simulink model "wlan_phy_rx_pmd"  (Simulink 7.8)
settings: Solver=VariableStepDiscrete  SolverMode=SingleTasking  StartTime=0.0  StopTime=rx_sim_time  RelTol=1e-3
PostLoadFcn:
  | wlan_phy_rx_init
InitFcn:
  | wlan_phy_rx_init
StartFcn:
  | wlan_phy_rx_init
values from neighbouring files (symbols this model uses):
  barker_seq20 = 0.95 * circshift(barker_seq20, [0 4]) ./ max(abs(barker_seq20))    [wlan_phy_rx_init.m]
  bit_scrambler_lfsr_bytes = sum(bit_scrambler_lfsr_bytes .* repmat(2.^[0:7], 127, 1),2)    [wlan_phy_rx_init.m]
  deinterleave_ROM = [deinterleave_ROM interleave_11n_64QAM zeros(1, 512-length(interleave_11n_64QAM))]    [wlan_phy_rx_init.m]
  longCorr_coef_i = [3*real(longCorr_coef)]    [wlan_phy_rx_init.m]
  longCorr_coef_q = [3*imag(longCorr_coef)]    [wlan_phy_rx_init.m]
  MAX_CP_LEN = 32    [wlan_phy_rx_init.m]
  MAX_NCBPS = NCBPS_11n_64QAM    (= 312)    [wlan_phy_rx_init.m]
  MAX_NUM_BYTES = 2^16-1    (= 65535)    [wlan_phy_rx_init.m]
  MAX_NUM_SC = 64    [wlan_phy_rx_init.m]
  MAX_NUM_SYMS = 600    [wlan_phy_rx_init.m]
  NCBPS_11a_BPSK = 48    [wlan_phy_rx_init.m]
  NDBPS_11a_BPSK12 = 24    [wlan_phy_rx_init.m]
  PHY_SIGNAL_MIN_LEN = 14    [wlan_phy_rx_init.m]
  preFFT_sampBuff_numSamps = 4*MAX_NUM_SC    (= 256)    [wlan_phy_rx_init.m]
  rx_sim_sig_samp_time = 8    [wlan_phy_rx_init.m]
  scr_ind_rev = zeros(1,128)    [wlan_phy_rx_init.m]
undefined symbols (not defined in the file or in its folder): gcbh, mcs_rom_11ag, mcs_rom_11n, xlBlockLoadCallback, xlGetNormalizedPeriod, xlipgetpartsetting
libraries: xbsIndex_r4 x3862, simulink x1, xbsEDKLib_r4 x1
(part 9 of 23)

== system wlan_phy_rx_pmd/DSSS Rx/Demod/DBPSK Demod ==
  IQ Valid : Inport
  I : Inport  Port=2
  Q : Inport  Port=3
  Absolute : xbsIndex_r4/Absolute  precision=Full  arith_type=Unsigned  n_bits=16  bin_pt=14  quantization=Truncate  overflow=Wrap  en=off  latency=0  dbl_ovrd=off  block_type=abs
  AddSub : xbsIndex_r4/AddSub  mode=Subtraction  use_carryin=off  use_carryout=off  en=off  latency=0  precision=Full  arith_type=Unsigned  n_bits=16  bin_pt=14  quantization=Truncate  overflow=Wrap  dbl_ovrd=off  use_behavioral_HDL=off  hw_selection=Fabric  pipelined=off  block_type=addsub
  "CORDIC 5.0 " : xbsIndex_r4/CORDIC 5.0   functional_selection=Arc_Tan  architectural_configuration=Parallel  pipelining_mode=Maximum  data_format=SignedFraction  phase_format=Scaled_Radians  input_width=16  output_width=16  round_mode=Truncate  iterations=0  precision=0  compensation_scaling=No_Scale_Compensation  coarse_rotation=on  aclken=off  aresetn=off  out_tready=off  cartesian_has_tuser=off  cartesian_has_tlast=off  cartesian_tuser_width=1  phase_has_tuser=off  phase_has_tlast=off  phase_tuser_width=1  out_tlast_behv=Null  flow_control=NonBlocking  optimize_goal=Resources  trim_axipin_name=on  ip_name=CORDIC  ip_version=5.0  dsptool_ready=true  ipcore_usecache=true  ipcore_useipmodelcache=true  wrapper_available=true  port_translation_map={ 'aclken' => 'en', 'aresetn' => 'rst' }  block_type=cordic_v5_0
      settings: infoedit=CORDIC 5.0
  Constant : xbsIndex_r4/Constant  const=0.5  gui_display_data_type=Fixed-point  arith_type=Signed (2's comp)  n_bits=4  bin_pt=3  preci_type=Single  exp_width=8  frac_width=24  explicit_period=off  period=1  equ=P=C  opselect=C  inp2=PCIN>>17  opr=+  inp1=P  carry=CIN  dbl_ovrd=off  block_type=constant
  Constant1 : xbsIndex_r4/Constant  const=1.5  gui_display_data_type=Fixed-point  arith_type=Signed (2's comp)  n_bits=6  bin_pt=3  preci_type=Single  exp_width=8  frac_width=24  explicit_period=off  period=1  equ=P=C  opselect=C  inp2=PCIN>>17  opr=+  inp1=P  carry=CIN  dbl_ovrd=off  block_type=constant
  Convert : xbsIndex_r4/Convert  gui_display_data_type=Fixed-point  arith_type=Unsigned  n_bits=1  bin_pt=0  float_type=Single  exp_bits=8  fraction_bits=24  quantization=Truncate  overflow=Wrap  en=off  latency=0  dbl_ovrd=off  pipeline=off  block_type=convert
  Gateway Out2 : xbsIndex_r4/Gateway Out  inherit_from_input=off  hdl_port=off  timing_constraint=None  locs_specified=off  LOCs={}  UseAsDAC=off  DACChannel='1'  block_type=gatewayout
  Gateway Out3 : xbsIndex_r4/Gateway Out  inherit_from_input=off  hdl_port=off  timing_constraint=None  locs_specified=off  LOCs={}  UseAsDAC=off  DACChannel='1'  block_type=gatewayout
  Gateway Out6 : xbsIndex_r4/Gateway Out  inherit_from_input=off  hdl_port=off  timing_constraint=None  locs_specified=off  LOCs={}  UseAsDAC=off  DACChannel='1'  block_type=gatewayout
  Logical : xbsIndex_r4/Logical  logical_function=AND  inputs=2  en=off  latency=0  precision=Full  arith_type=Unsigned  n_bits=16  bin_pt=0  align_bp=on  dbl_ovrd=off  block_type=logical
  Phase Cmp : Scope  NumInputPorts=7
      settings: TimeRange=80000  YMin=-1~-0.5~0~-0.1~5~0~0  YMax=1~0.3~1~1.1~40~1~300  SaveName=ScopeData6
  Register{,1..3} (4 alike) : xbsIndex_r4/Register  init=0  rst=off  en=off  dbl_ovrd=off  block_type=register
  Register4 : xbsIndex_r4/Register  init=0  rst=off  en=on  dbl_ovrd=off  block_type=register
  Relational : xbsIndex_r4/Relational  mode=a>b  en=off  latency=0  dbl_ovrd=off  block_type=relational
  Relational1 : xbsIndex_r4/Relational  mode=a<b  en=off  latency=0  dbl_ovrd=off  block_type=relational
  bit : Outport
  bit valid : Outport  Port=2
  wires:
  IQ Valid/1 -> "CORDIC 5.0 "/1
  I/1 -> "CORDIC 5.0 "/2
  Q/1 -> "CORDIC 5.0 "/3
  "CORDIC 5.0 "/2 -> Register1/1
  "CORDIC 5.0 "/1 -> Register/1
  Register4/1 -> AddSub/2, Gateway Out3/1
  AddSub/1 -> Absolute/1
  Constant/1 -> Relational/2
  Absolute/1 -> Gateway Out6/1, Relational/1, Relational1/1
  Relational/1 -> Logical/1
  Convert/1 -> Register2/1
  Gateway Out2/1 -> Phase Cmp/1
  Gateway Out3/1 -> Phase Cmp/2
  Gateway Out6/1 -> Phase Cmp/3
  Constant1/1 -> Relational1/2
  Relational1/1 -> Logical/2
  Logical/1 -> Convert/1
  Register/1 -> Register3/1, Register4/2
  Register1/1 -> AddSub/1, Gateway Out2/1, Register4/1
  Register2/1 -> bit/1
  Register3/1 -> bit valid/1

== system wlan_phy_rx_pmd/DSSS Rx/Demod/De-spread ==
  I : Inport
  Q : Inport  Port=2
  Sym Start : Inport  Port=3
  Accumulator : xbsIndex_r4/Accumulator  operation=Add  n_bits=22  overflow=Wrap  scale=1  rst=on  infoeditControl=reset for floating point data type must be asserted for a minimum of 2 cycles  hasbypass=on  en=off  latency=0  dbl_ovrd=off  msb_inp=100  msb=100  lsb=-100  use_behavioral_HDL=on  implementation=Fabric  block_type=accum
  Accumulator1 : xbsIndex_r4/Accumulator  operation=Add  n_bits=22  overflow=Wrap  scale=1  rst=on  infoeditControl=reset for floating point data type must be asserted for a minimum of 2 cycles  hasbypass=on  en=off  latency=0  dbl_ovrd=off  msb_inp=100  msb=100  lsb=-100  use_behavioral_HDL=on  implementation=Fabric  block_type=accum
  Constant : xbsIndex_r4/Constant  const=0  gui_display_data_type=Fixed-point  arith_type=Unsigned  n_bits=2  bin_pt=0  preci_type=Single  exp_width=8  frac_width=24  explicit_period=off  period=1  equ=P=C  opselect=C  inp2=PCIN>>17  opr=+  inp1=P  carry=CIN  dbl_ovrd=off  block_type=constant
  Constant1 : xbsIndex_r4/Constant  const=0  gui_display_data_type=Boolean  arith_type=Boolean  n_bits=16  bin_pt=14  preci_type=Single  exp_width=8  frac_width=24  explicit_period=on  period=8  equ=P=C  opselect=C  inp2=PCIN>>17  opr=+  inp1=P  carry=CIN  dbl_ovrd=off  block_type=constant
  Convert : xbsIndex_r4/Convert  gui_display_data_type=Fixed-point  arith_type="Signed  (2's comp)"  n_bits=16  bin_pt=14  float_type=Single  exp_bits=8  fraction_bits=24  quantization=Truncate  overflow=Saturate  en=off  latency=0  dbl_ovrd=off  pipeline=off  block_type=convert
  Convert1 : xbsIndex_r4/Convert  gui_display_data_type=Fixed-point  arith_type="Signed  (2's comp)"  n_bits=16  bin_pt=14  float_type=Single  exp_bits=8  fraction_bits=24  quantization=Truncate  overflow=Saturate  en=off  latency=0  dbl_ovrd=off  pipeline=off  block_type=convert
  Counter : xbsIndex_r4/Counter  cnt_type=Count Limited  cnt_to=19  operation=Up  start_count=0  cnt_by_val=1  arith_type=Unsigned  n_bits=5  bin_pt=0  load_pin=off  rst=on  en=on  explicit_period=off  period=1  dbl_ovrd=off  use_behavioral_HDL=off  implementation=Fabric  block_type=counter
  Delay : xbsIndex_r4/Delay  rst=off  infoeditControl=Selection of Reset will increase slice count due to use of real FFs and instead of SRLs  en=off  latency=1  dbl_ovrd=off  reg_retiming=off  block_type=delay
  Gateway Out{1..9} (9 alike) : xbsIndex_r4/Gateway Out  inherit_from_input=off  hdl_port=off  timing_constraint=None  locs_specified=off  LOCs={}  UseAsDAC=off  DACChannel='1'  block_type=gatewayout
  Inverter : xbsIndex_r4/Inverter  en=off  latency=0  dbl_ovrd=off  block_type=inv
  Logical1 : xbsIndex_r4/Logical  logical_function=AND  inputs=2  en=off  latency=0  precision=Full  arith_type=Unsigned  n_bits=8  bin_pt=2  align_bp=on  dbl_ovrd=off  block_type=logical
      settings: xl_area=[1 0 0 1 0 0 0]  block_version=VER_STRING_GOES_HERE
  Mult : xbsIndex_r4/Mult  precision=User Defined  arith_type="Signed  (2's comp)"  n_bits=16  bin_pt=15  quantization=Truncate  overflow=Wrap  en=off  latency=1  dbl_ovrd=off  use_behavioral_HDL=off  opt=Speed  use_embedded=on  optimum_pipeline=off  pipeline=on  use_rpm=on  placement_style=Triangular  block_type=mult
  Mult1 : xbsIndex_r4/Mult  precision=User Defined  arith_type="Signed  (2's comp)"  n_bits=16  bin_pt=15  quantization=Truncate  overflow=Wrap  en=off  latency=1  dbl_ovrd=off  use_behavioral_HDL=off  opt=Speed  use_embedded=on  optimum_pipeline=off  pipeline=on  use_rpm=on  placement_style=Triangular  block_type=mult
  ROM : xbsIndex_r4/ROM  depth=length(barker_seq20)  initVector=fliplr(barker_seq20)  distributed_mem=Block RAM  rst=off  init_reg=0  en=off  latency=1  gui_display_data_type=Fixed-point  arith_type="Signed  (2's comp)"  n_bits=16  bin_pt=15  preci_type=Single  exp_width=8  frac_width=24  dbl_ovrd=off  optimize=Area  use_rpm=on  block_type=sprom
  Real-Imag to Complex : RealImagToComplex
  Register : xbsIndex_r4/Register  init=0  rst=on  en=on  dbl_ovrd=off  block_type=register
  Register1 : xbsIndex_r4/Register  init=0  rst=on  en=on  dbl_ovrd=off  block_type=register
  Register2 : xbsIndex_r4/Register  init=0  rst=on  en=off  dbl_ovrd=off  block_type=register
  Register3 : xbsIndex_r4/Register  init=0  rst=on  en=off  dbl_ovrd=off  block_type=register
  Register{4..7} (4 alike) : xbsIndex_r4/Register  init=0  rst=off  en=off  dbl_ovrd=off  block_type=register
  Relational : xbsIndex_r4/Relational  mode=a=b  en=off  latency=0  dbl_ovrd=off  block_type=relational
  Scope : Scope  NumInputPorts=7
      settings: TimeRange=60000  YMin=-1~-1~-1~-1~-0.4~-0.5~0  YMax=1~1~0.5~1~0.4~1~1  SaveName=ScopeData4
  To Workspace : ToWorkspace  VariableName=simout  SampleTime=-1  SaveFormat=Array
  IQ Valid : Outport
  " I" : Outport  Port=2
  " Q" : Outport  Port=3
  tag scope: CS_DSSS_Despread_I=global, CS_DSSS_Despread_Q=global
  wires:
  I/1 -> Register4/1
  Counter/1 -> ROM/1, Relational/1
  ROM/1 -> Register6/1
  Mult/1 -> Accumulator/1
  Mult1/1 -> Accumulator1/1
  Constant/1 -> Relational/2
  Relational/1 -> Register7/1
  Inverter/1 -> Counter/1, Register3/2, Register2/2, Register/2, Register1/2
  Accumulator/1 -> Register/1, Gateway Out8/1
  Accumulator1/1 -> Register1/1, Gateway Out9/1
  Real-Imag to Complex/1 -> To Workspace/1
  Register2/1 -> Gateway Out2/1
  Register3/1 -> Gateway Out3/1
  Gateway Out2/1 -> Real-Imag to Complex/1
  Gateway Out3/1 -> Real-Imag to Complex/2
  Register/1 -> Convert/1, Gateway Out1/1
  Q/1 -> Register5/1
  Register1/1 -> Convert1/1, Gateway Out6/1
  Delay/1 -> Logical1/1
  Sym Start/1 -> Logical1/2, Inverter/1, Gateway Out7/1, Counter/2
  Convert/1 -> " I"/1, [CS_DSSS_Despread_I]
  Convert1/1 -> " Q"/1, [CS_DSSS_Despread_Q]
  Logical1/1 -> IQ Valid/1
  Gateway Out4/1 -> Scope/1
  Gateway Out5/1 -> Scope/2
  Gateway Out8/1 -> Scope/3
  Gateway Out9/1 -> Scope/4
  Gateway Out1/1 -> Scope/5
  Gateway Out6/1 -> Scope/6
  Register4/1 -> Mult/1, Register2/1, Gateway Out4/1
  Register5/1 -> Mult1/1
  Register6/1 -> Mult1/2, Mult/2, Register3/1, Gateway Out5/1
  Register7/1 -> Accumulator1/2, Register1/3, Register/3, Delay/1, Accumulator/2
  Gateway Out7/1 -> Scope/7

== system wlan_phy_rx_pmd/DSSS Rx/Demod/Descramble ==
  Bit : Inport
  Bit Valid : Inport  Port=2
  Convert : xbsIndex_r4/Convert  gui_display_data_type=Boolean  arith_type=Boolean  n_bits=16  bin_pt=14  float_type=Single  exp_bits=8  fraction_bits=24  quantization=Truncate  overflow=Wrap  en=off  latency=0  dbl_ovrd=off  pipeline=off  block_type=convert
  Convert1 : xbsIndex_r4/Convert  gui_display_data_type=Boolean  arith_type=Boolean  n_bits=16  bin_pt=14  float_type=Single  exp_bits=8  fraction_bits=24  quantization=Truncate  overflow=Wrap  en=off  latency=0  dbl_ovrd=off  pipeline=off  block_type=convert
  Convert2 : xbsIndex_r4/Convert  gui_display_data_type=Fixed-point  arith_type=Unsigned  n_bits=1  bin_pt=0  float_type=Single  exp_bits=8  fraction_bits=24  quantization=Truncate  overflow=Wrap  en=off  latency=0  dbl_ovrd=off  pipeline=off  block_type=convert
  Logical : xbsIndex_r4/Logical  logical_function=XOR  inputs=2  en=off  latency=0  precision=Full  arith_type=Unsigned  n_bits=8  bin_pt=2  align_bp=on  dbl_ovrd=off  block_type=logical
      settings: xl_area=[0, 0, 0, 0, 0, 0, 0]  block_version=VER_STRING_GOES_HERE
  Logical1 : xbsIndex_r4/Logical  logical_function=XOR  inputs=2  en=off  latency=0  precision=Full  arith_type=Unsigned  n_bits=8  bin_pt=2  align_bp=on  dbl_ovrd=off  block_type=logical
      settings: xl_area=[0, 0, 0, 0, 0, 0, 0]  block_version=VER_STRING_GOES_HERE
  Register{,1..6} (7 alike) : xbsIndex_r4/Register  init=1  rst=on  en=on  dbl_ovrd=off  block_type=register
      settings: xl_area=[0, 0, 0, 0, 0, 0, 0]  block_version=VER_STRING_GOES_HERE
  Register7 : xbsIndex_r4/Register  init=0  rst=off  en=off  dbl_ovrd=off  block_type=register
  Register8 : xbsIndex_r4/Register  init=0  rst=off  en=off  dbl_ovrd=off  block_type=register
  " Bit" : Outport
  " Bit Valid" : Outport  Port=2
  wires:
  [DSSS_RX_RESET] -> Convert/1
  Register6/1 -> Logical/1
  Register5/1 -> Register6/1
  Register4/1 -> Register5/1
  Register3/1 -> Register4/1, Logical/2
  Register2/1 -> Register3/1
  Register1/1 -> Register2/1
  Register/1 -> Register1/1
  Bit Valid/1 -> Convert1/1
  Convert/1 -> Register5/2, Register6/2, Register4/2, Register3/2, Register2/2, Register1/2, Register/2
  Convert1/1 -> Register5/3, Register6/3, Register8/1, Register4/3, Register3/3, Register2/3, Register1/3, Register/3
  Convert2/1 -> Register7/1
  Bit/1 -> Register/1, Logical1/1
  Logical/1 -> Logical1/2
  Logical1/1 -> Convert2/1
  Register7/1 -> " Bit"/1
  Register8/1 -> " Bit Valid"/1

== system wlan_phy_rx_pmd/DSSS Rx/Demod/Header CRC ==
  Bit : Inport
  Bit Valid : Inport  Port=2
  CRC LFSR : Subsystem
  Constant : xbsIndex_r4/Constant  const=32  gui_display_data_type=Fixed-point  arith_type=Unsigned  n_bits=6  bin_pt=0  preci_type=Single  exp_width=8  frac_width=24  explicit_period=off  period=1  equ=P=C  opselect=C  inp2=PCIN>>17  opr=+  inp1=P  carry=CIN  dbl_ovrd=off  block_type=constant
  Counter1 : xbsIndex_r4/Counter  cnt_type=Free Running  cnt_to=Inf  operation=Up  start_count=0  cnt_by_val=1  arith_type=Unsigned  n_bits=8  bin_pt=0  load_pin=off  rst=on  en=on  explicit_period=off  period=1  dbl_ovrd=off  use_behavioral_HDL=off  implementation=Fabric  block_type=counter
  Display : Display
  Gateway Out{1..6} (6 alike) : xbsIndex_r4/Gateway Out  inherit_from_input=off  hdl_port=off  timing_constraint=None  locs_specified=off  LOCs={}  UseAsDAC=off  DACChannel='1'  block_type=gatewayout
  Logical1 : xbsIndex_r4/Logical  logical_function=AND  inputs=2  en=off  latency=0  precision=Full  arith_type=Unsigned  n_bits=16  bin_pt=0  align_bp=on  dbl_ovrd=off  block_type=logical
  Register8 : xbsIndex_r4/Register  init=0  rst=off  en=on  dbl_ovrd=off  block_type=register
  Relational1 : xbsIndex_r4/Relational  mode=a<b  en=off  latency=0  dbl_ovrd=off  block_type=relational
  Scope : Scope  NumInputPorts=7
      settings: TimeRange=100000  YMin=-1~-1~-1~-0.5~0~0~0  YMax=1~1~1~1.5~150~1~1  SaveName=ScopeData3
  CRC Calc : Outport
  wires:
  [DSSS_RX_RESET] -> Counter1/1, Gateway Out6/1
  Counter1/1 -> Relational1/1, Gateway Out2/1
  Constant/1 -> Relational1/2
  Relational1/1 -> Logical1/2
  Bit/1 -> CRC LFSR/1(Bit), Register8/1
  CRC LFSR/1(CRC16) -> Gateway Out4/1, CRC Calc/1
  Gateway Out4/1 -> Display/1
  Gateway Out1/1 -> Scope/6
  Gateway Out5/1 -> Scope/7
  Gateway Out2/1 -> Scope/5
  Gateway Out3/1 -> Scope/4
  Register8/1 -> Gateway Out3/1
  Gateway Out6/1 -> Scope/1
  Bit Valid/1 -> Logical1/1, Gateway Out1/1
  Logical1/1 -> Counter1/2, CRC LFSR/2(Bit Valid), Gateway Out5/1, Register8/2

== system wlan_phy_rx_pmd/DSSS Rx/Demod/Sync ==
  note: Timeout in two stages:
      |
      | A) In the first MIN_ONES_TOT bits, at least MIN_ONES bits must be 1 (the DSSS preamble is 128 1's).
      |   Timeout and reset if this fails.
      |
      | -If (A) is satisfied, search for the SFD magic number in the first BITS_TO_SFD bits. Timeout and reset
      |   if no SFD is found.
  Bit : Inport
  Bit Valid : Inport  Port=2
  Convert1 : xbsIndex_r4/Convert  gui_display_data_type=Boolean  arith_type=Boolean  n_bits=1  bin_pt=0  float_type=Single  exp_bits=8  fraction_bits=24  quantization=Truncate  overflow=Wrap  en=off  latency=0  dbl_ovrd=off  pipeline=off  block_type=convert
  Convert2 : xbsIndex_r4/Convert  gui_display_data_type=Boolean  arith_type=Boolean  n_bits=1  bin_pt=0  float_type=Single  exp_bits=8  fraction_bits=24  quantization=Truncate  overflow=Wrap  en=off  latency=0  dbl_ovrd=off  pipeline=off  block_type=convert
  Counter1 : xbsIndex_r4/Counter  cnt_type=Free Running  cnt_to=Inf  operation=Up  start_count=0  cnt_by_val=1  arith_type=Unsigned  n_bits=8  bin_pt=0  load_pin=off  rst=on  en=on  explicit_period=off  period=1  dbl_ovrd=off  use_behavioral_HDL=off  implementation=Fabric  block_type=counter
  Counter2 : xbsIndex_r4/Counter  cnt_type=Free Running  cnt_to=Inf  operation=Up  start_count=0  cnt_by_val=1  arith_type=Unsigned  n_bits=8  bin_pt=0  load_pin=off  rst=on  en=on  explicit_period=off  period=1  dbl_ovrd=off  use_behavioral_HDL=off  implementation=Fabric  block_type=counter
  DSSS Sync : Scope  NumInputPorts=7
      settings: TimeRange=56900  YMin=0~0~0~0~0~-1~-1  YMax=1~1~1~70~45~1~1  SaveName=ScopeData2
  Display : Display
  Display1 : Display
  Display2 : Display
  Down Sample1 : xbsIndex_r4/Down Sample  sample_ratio=8  sample_phase="Last Value of Frame  (most efficient)"  en=off  latency=1  dbl_ovrd=off  block_type=dsamp
  Gateway Out{,1..9} (10 alike) : xbsIndex_r4/Gateway Out  inherit_from_input=off  hdl_port=off  timing_constraint=None  locs_specified=off  LOCs={}  UseAsDAC=off  DACChannel='1'  block_type=gatewayout
  Inverter : xbsIndex_r4/Inverter  en=off  latency=0  dbl_ovrd=off  block_type=inv
      settings: xl_area=[0 0 0 0 0 0 0]  block_version=VER_STRING_GOES_HERE
  Logical : xbsIndex_r4/Logical  logical_function=AND  inputs=2  en=off  latency=0  precision=Full  arith_type=Unsigned  n_bits=8  bin_pt=2  align_bp=on  dbl_ovrd=off  block_type=logical
      settings: xl_area=[0, 0, 0, 0, 0, 0, 0]  block_version=VER_STRING_GOES_HERE
  Logical1 : xbsIndex_r4/Logical  logical_function=AND  inputs=3  en=off  latency=0  precision=Full  arith_type=Unsigned  n_bits=8  bin_pt=2  align_bp=on  dbl_ovrd=off  block_type=logical
      settings: xl_area=[0, 0, 0, 0, 0, 0, 0]  block_version=VER_STRING_GOES_HERE
  Logical2 : xbsIndex_r4/Logical  logical_function=AND  inputs=2  en=off  latency=0  precision=Full  arith_type=Unsigned  n_bits=8  bin_pt=2  align_bp=on  dbl_ovrd=off  block_type=logical
      settings: xl_area=[0, 0, 0, 0, 0, 0, 0]  block_version=VER_STRING_GOES_HERE
  Logical3 : xbsIndex_r4/Logical  logical_function=OR  inputs=2  en=off  latency=0  precision=Full  arith_type=Unsigned  n_bits=8  bin_pt=2  align_bp=on  dbl_ovrd=off  block_type=logical
      settings: xl_area=[0, 0, 0, 0, 0, 0, 0]  block_version=VER_STRING_GOES_HERE
  Logical4 : xbsIndex_r4/Logical  logical_function=AND  inputs=2  en=off  latency=0  precision=Full  arith_type=Unsigned  n_bits=8  bin_pt=2  align_bp=on  dbl_ovrd=off  block_type=logical
      settings: xl_area=[0, 0, 0, 0, 0, 0, 0]  block_version=VER_STRING_GOES_HERE
  Register8 : xbsIndex_r4/Register  init=0  rst=off  en=on  dbl_ovrd=off  block_type=register
  Relational1 : xbsIndex_r4/Relational  mode=a>b  en=off  latency=0  dbl_ovrd=off  block_type=relational
  Relational2 : xbsIndex_r4/Relational  mode=a>b  en=off  latency=0  dbl_ovrd=off  block_type=relational
  Relational3 : xbsIndex_r4/Relational  mode=a>b  en=off  latency=0  dbl_ovrd=off  block_type=relational
  S-R Latch : Subsystem
  SFD Search : Subsystem
  Payload Bit : Outport
  " Bit Valid" : Outport  Port=2
  tag scope: DSSS_RX_TIMEOUT=global
  wires:
  Bit/1 -> SFD Search/1(Bit), Convert1/1, Payload Bit/1, Register8/1
  Bit Valid/1 -> SFD Search/2(Bit Valid), Logical/2, Counter1/2, Convert2/1, Gateway Out1/1, Register8/2
  SFD Search/1(Found) -> S-R Latch/1(S)
  [DSSS_RX_RESET] -> S-R Latch/2(R)
  S-R Latch/1(Q) -> Logical/1, Inverter/1, Gateway Out4/1
  Logical/1 -> " Bit Valid"/1, Gateway Out9/1
  Counter1/1 -> Relational1/1, Relational3/1, Gateway Out5/1
  Gateway Out/1 -> Display/1
  [DSSS_RX_RESET] -> Counter1/1, Counter2/1
  Gateway Out4/1 -> DSSS Sync/3  "SFD Found"
  Gateway Out5/1 -> DSSS Sync/4  "Bit Counter"
  Gateway Out1/1 -> DSSS Sync/2  "Bit Valid"
  Down Sample1/1 -> Relational1/2
  Gateway Out2/1 -> DSSS Sync/1  "Bit"
  Logical2/1 -> Counter2/2
  [regRx_DSSS_RX_TIMEOUT_BITS_TO_SFD] -> Down Sample1/1
  Counter2/1 -> Relational2/2, Gateway Out7/1
  [regRx_DSSS_RX_TIMEOUT_MIN_ONES] -> Relational2/1
  [regRx_DSSS_RX_TIMEOUT_MIN_ONES_TOT] -> Relational3/2
  Relational3/1 -> Logical1/2
  Relational2/1 -> Logical1/3
  Logical3/1 -> [DSSS_RX_TIMEOUT], Gateway Out8/1
  Inverter/1 -> Logical1/1, Logical4/1
  Logical1/1 -> Logical3/2
  [regRx_DSSS_RX_TIMEOUT_BITS_TO_SFD] -> Gateway Out/1
  Gateway Out3/1 -> Display1/1
  [regRx_DSSS_RX_TIMEOUT_MIN_ONES_TOT] -> Gateway Out3/1
  Gateway Out6/1 -> Display2/1
  [regRx_DSSS_RX_TIMEOUT_MIN_ONES] -> Gateway Out6/1
  Gateway Out7/1 -> DSSS Sync/5  "Ones Counter"
  Gateway Out8/1 -> DSSS Sync/6  "Timed Out"
  Convert2/1 -> Logical2/1
  Convert1/1 -> Logical2/2
  Register8/1 -> Gateway Out2/1
  Logical4/1 -> Logical3/1
  Relational1/1 -> Logical4/2
  Gateway Out9/1 -> DSSS Sync/7  "Bit Valid"

== system wlan_phy_rx_pmd/DSSS Rx/Reset Gen/Posedge ==
  D : Inport
  Delay : xbsIndex_r4/Delay  rst=off  infoeditControl=Selection of Reset will increase slice count due to use of real FFs and instead of SRLs  en=off  latency=1  dbl_ovrd=off  reg_retiming=off  block_type=delay
      settings: infoedit=Hardware notes: A delay line is a chain, each link of which is an SRL16 followed by a flip-flop. If register retiming is enabled, the delay line is a chain of flip-flops.  xl_area=[1 1 0 0 0 0 0]  block_version=VER_STRING_GOES_HERE
  Inverter : xbsIndex_r4/Inverter  en=off  latency=0  dbl_ovrd=off  block_type=inv
      settings: xl_area=[0 0 0 0 0 0 0]  block_version=VER_STRING_GOES_HERE
  Logical1 : xbsIndex_r4/Logical  logical_function=AND  inputs=2  en=off  latency=0  precision=Full  arith_type=Unsigned  n_bits=8  bin_pt=2  align_bp=on  dbl_ovrd=off  block_type=logical
      settings: xl_area=[1 0 0 1 0 0 0]  block_version=VER_STRING_GOES_HERE
  Q : Outport
  wires:
  D/1 -> Logical1/2, Delay/1
  Logical1/1 -> Q/1
  Inverter/1 -> Logical1/1
  Delay/1 -> Inverter/1

== system wlan_phy_rx_pmd/DSSS Rx/Reset Gen/S-R Latch ==
  S : Inport
  R : Inport  Port=2
  Constant1 : xbsIndex_r4/Constant  const=1  gui_display_data_type=Boolean  arith_type=Boolean  n_bits=1  bin_pt=0  preci_type=Single  exp_width=8  frac_width=24  explicit_period=off  period=1  equ=P=C  opselect=C  inp2=PCIN>>17  opr=+  inp1=P  carry=CIN  dbl_ovrd=off  block_type=constant
      settings: block_version=VER_STRING_GOES_HERE
  Convert1 : xbsIndex_r4/Convert  gui_display_data_type=Boolean  arith_type=Boolean  n_bits=1  bin_pt=0  float_type=Single  exp_bits=8  fraction_bits=24  quantization=Truncate  overflow=Wrap  en=off  latency=0  dbl_ovrd=off  pipeline=off  block_type=convert
  Convert2 : xbsIndex_r4/Convert  gui_display_data_type=Boolean  arith_type=Boolean  n_bits=1  bin_pt=0  float_type=Single  exp_bits=8  fraction_bits=24  quantization=Truncate  overflow=Wrap  en=off  latency=0  dbl_ovrd=off  pipeline=off  block_type=convert
  Register2 : xbsIndex_r4/Register  init=0  rst=on  en=on  dbl_ovrd=off  block_type=register
      settings: xl_area=[1 1 0 0 0 0 0]  block_version=VER_STRING_GOES_HERE
  Q : Outport
  wires:
  Convert2/1 -> Register2/3
  Convert1/1 -> Register2/2
  Constant1/1 -> Register2/1
  R/1 -> Convert1/1
  Register2/1 -> Q/1
  S/1 -> Convert2/1

== system wlan_phy_rx_pmd/DSSS Rx/Reset Gen/S-R Latch2 ==
  S : Inport
  R : Inport  Port=2
  Constant1 : xbsIndex_r4/Constant  const=1  gui_display_data_type=Boolean  arith_type=Boolean  n_bits=1  bin_pt=0  preci_type=Single  exp_width=8  frac_width=24  explicit_period=off  period=1  equ=P=C  opselect=C  inp2=PCIN>>17  opr=+  inp1=P  carry=CIN  dbl_ovrd=off  block_type=constant
      settings: block_version=VER_STRING_GOES_HERE
  Convert1 : xbsIndex_r4/Convert  gui_display_data_type=Boolean  arith_type=Boolean  n_bits=1  bin_pt=0  float_type=Single  exp_bits=8  fraction_bits=24  quantization=Truncate  overflow=Wrap  en=off  latency=0  dbl_ovrd=off  pipeline=off  block_type=convert
  Convert2 : xbsIndex_r4/Convert  gui_display_data_type=Boolean  arith_type=Boolean  n_bits=1  bin_pt=0  float_type=Single  exp_bits=8  fraction_bits=24  quantization=Truncate  overflow=Wrap  en=off  latency=0  dbl_ovrd=off  pipeline=off  block_type=convert
  Register2 : xbsIndex_r4/Register  init=0  rst=on  en=on  dbl_ovrd=off  block_type=register
      settings: xl_area=[1 1 0 0 0 0 0]  block_version=VER_STRING_GOES_HERE
  Q : Outport
  wires:
  S/1 -> Convert2/1
  Register2/1 -> Q/1
  R/1 -> Convert1/1
  Constant1/1 -> Register2/1
  Convert1/1 -> Register2/2
  Convert2/1 -> Register2/3

== system wlan_phy_rx_pmd/DSSS Rx/Reset Gen/negedge ==
  D : Inport
  Delay : xbsIndex_r4/Delay  rst=off  infoeditControl=Selection of Reset will increase slice count due to use of real FFs and instead of SRLs  en=off  latency=1  dbl_ovrd=off  reg_retiming=off  block_type=delay
      settings: infoedit=Hardware notes: A delay line is a chain, each link of which is an SRL16 followed by a flip-flop. If register retiming is enabled, the delay line is a chain of flip-flops.  xl_area=[1 1 0 0 0 0 0]  block_version=VER_STRING_GOES_HERE
  Inverter : xbsIndex_r4/Inverter  en=off  latency=0  dbl_ovrd=off  block_type=inv
      settings: xl_area=[0 0 0 0 0 0 0]  block_version=VER_STRING_GOES_HERE
  Logical1 : xbsIndex_r4/Logical  logical_function=AND  inputs=2  en=off  latency=0  precision=Full  arith_type=Unsigned  n_bits=8  bin_pt=2  align_bp=on  dbl_ovrd=off  block_type=logical
      settings: xl_area=[1 0 0 1 0 0 0]  block_version=VER_STRING_GOES_HERE
  Q : Outport
  wires:
  Delay/1 -> Logical1/1
  Logical1/1 -> Q/1
  D/1 -> Delay/1, Inverter/1
  Inverter/1 -> Logical1/2

== system wlan_phy_rx_pmd/DSSS Rx/SIGNAL Dec/Length Check ==
  note: Require [14,1500] bytes - reject anything else
      | as invalid. Reduces wasted time attempting
      | decoding of bogus LENGTH pkts.
  Len : Inport
  Constant1 : xbsIndex_r4/Constant  const=8*14  gui_display_data_type=Fixed-point  arith_type=Unsigned  n_bits=8  bin_pt=0  preci_type=Single  exp_width=8  frac_width=24  explicit_period=off  period=1  equ=P=C  opselect=C  inp2=PCIN>>17  opr=+  inp1=P  carry=CIN  dbl_ovrd=off  block_type=constant
  Constant5 : xbsIndex_r4/Constant  const=8*1500  gui_display_data_type=Fixed-point  arith_type=Unsigned  n_bits=14  bin_pt=0  preci_type=Single  exp_width=8  frac_width=24  explicit_period=off  period=1  equ=P=C  opselect=C  inp2=PCIN>>17  opr=+  inp1=P  carry=CIN  dbl_ovrd=off  block_type=constant
  Logical9 : xbsIndex_r4/Logical  logical_function=OR  inputs=2  en=off  latency=0  precision=Full  arith_type=Unsigned  n_bits=16  bin_pt=0  align_bp=on  dbl_ovrd=off  block_type=logical
  Relational1 : xbsIndex_r4/Relational  mode=a<b  en=off  latency=0  dbl_ovrd=off  block_type=relational
  Relational5 : xbsIndex_r4/Relational  mode=a>b  en=off  latency=0  dbl_ovrd=off  block_type=relational
  Invalid : Outport
  wires:
  Constant5/1 -> Relational5/2
  Len/1 -> Relational5/1, Relational1/1
  Relational5/1 -> Logical9/1
  Constant1/1 -> Relational1/2
  Relational1/1 -> Logical9/2
  Logical9/1 -> Invalid/1

== system wlan_phy_rx_pmd/DSSS Rx/Sym Sync/Abs ==
  Valid : Inport
  I : Inport  Port=2
  Q : Inport  Port=3
  AddSub : xbsIndex_r4/AddSub  mode=Addition  use_carryin=off  use_carryout=off  en=off  latency=1  precision=Full  arith_type=Unsigned  n_bits=16  bin_pt=14  quantization=Truncate  overflow=Wrap  dbl_ovrd=off  use_behavioral_HDL=off  hw_selection=Fabric  pipelined=off  block_type=addsub
  Inverter : xbsIndex_r4/Inverter  en=off  latency=0  dbl_ovrd=off  block_type=inv
  Mult : xbsIndex_r4/Mult  precision=Full  arith_type="Signed  (2's comp)"  n_bits=16  bin_pt=14  quantization=Truncate  overflow=Wrap  en=off  latency=1  dbl_ovrd=off  use_behavioral_HDL=off  opt=Speed  use_embedded=on  optimum_pipeline=off  pipeline=on  use_rpm=on  placement_style=Triangular  block_type=mult
  Mult1 : xbsIndex_r4/Mult  precision=Full  arith_type="Signed  (2's comp)"  n_bits=16  bin_pt=14  quantization=Truncate  overflow=Wrap  en=off  latency=1  dbl_ovrd=off  use_behavioral_HDL=off  opt=Speed  use_embedded=on  optimum_pipeline=off  pipeline=on  use_rpm=on  placement_style=Triangular  block_type=mult
  Register : xbsIndex_r4/Register  init=0  rst=on  en=off  dbl_ovrd=off  block_type=register
  A : Outport
  wires:
  I/1 -> Mult/2, Mult/1
  Mult/1 -> AddSub/1
  Mult1/1 -> AddSub/2
  AddSub/1 -> Register/1
  Q/1 -> Mult1/1, Mult1/2
  Valid/1 -> Inverter/1
  Register/1 -> A/1
  Inverter/1 -> Register/2

== system wlan_phy_rx_pmd/DSSS Rx/Sym Sync/Posedge ==
  D : Inport
  Delay : xbsIndex_r4/Delay  rst=off  infoeditControl=Selection of Reset will increase slice count due to use of real FFs and instead of SRLs  en=off  latency=1  dbl_ovrd=off  reg_retiming=off  block_type=delay
      settings: infoedit=Hardware notes: A delay line is a chain, each link of which is an SRL16 followed by a flip-flop. If register retiming is enabled, the delay line is a chain of flip-flops.  xl_area=[1 1 0 0 0 0 0]  block_version=VER_STRING_GOES_HERE
  Inverter : xbsIndex_r4/Inverter  en=off  latency=0  dbl_ovrd=off  block_type=inv
      settings: xl_area=[0 0 0 0 0 0 0]  block_version=VER_STRING_GOES_HERE
  Logical1 : xbsIndex_r4/Logical  logical_function=AND  inputs=2  en=off  latency=0  precision=Full  arith_type=Unsigned  n_bits=8  bin_pt=2  align_bp=on  dbl_ovrd=off  block_type=logical
      settings: xl_area=[1 0 0 1 0 0 0]  block_version=VER_STRING_GOES_HERE
  Q : Outport
  wires:
  D/1 -> Logical1/2, Delay/1
  Logical1/1 -> Q/1
  Inverter/1 -> Logical1/1
  Delay/1 -> Inverter/1

== system wlan_phy_rx_pmd/DSSS Rx/Sym Sync/S-R Latch ==
  S : Inport
  R : Inport  Port=2
  Constant1 : xbsIndex_r4/Constant  const=1  gui_display_data_type=Fixed-point  arith_type=Boolean  n_bits=1  bin_pt=0  preci_type=Single  exp_width=8  frac_width=24  explicit_period=off  period=1  equ=P=C  opselect=C  inp2=PCIN>>17  opr=+  inp1=P  carry=CIN  dbl_ovrd=off  block_type=constant
      settings: block_version=VER_STRING_GOES_HERE
  Convert1 : xbsIndex_r4/Convert  gui_display_data_type=Boolean  arith_type=Boolean  n_bits=1  bin_pt=0  float_type=Single  exp_bits=8  fraction_bits=24  quantization=Truncate  overflow=Wrap  en=off  latency=0  dbl_ovrd=off  pipeline=off  block_type=convert
  Convert2 : xbsIndex_r4/Convert  gui_display_data_type=Boolean  arith_type=Boolean  n_bits=1  bin_pt=0  float_type=Single  exp_bits=8  fraction_bits=24  quantization=Truncate  overflow=Wrap  en=off  latency=0  dbl_ovrd=off  pipeline=off  block_type=convert
  Register2 : xbsIndex_r4/Register  init=0  rst=on  en=on  dbl_ovrd=off  block_type=register
      settings: xl_area=[1 1 0 0 0 0 0]  block_version=VER_STRING_GOES_HERE
  Q : Outport
  wires:
  Convert2/1 -> Register2/3
  Convert1/1 -> Register2/2
  Constant1/1 -> Register2/1
  R/1 -> Convert1/1
  Register2/1 -> Q/1
  S/1 -> Convert2/1

== system wlan_phy_rx_pmd/DSSS Rx/Sym Sync/Sym Match ==
  Corr : Inport
  En : Inport  Port=2
  Delay{2..5} (4 alike) : xbsIndex_r4/Delay  rst=off  infoeditControl=Selection of Reset will increase slice count due to use of real FFs and instead of SRLs  en=off  latency=10  dbl_ovrd=off  reg_retiming=off  block_type=delay
  Inverter : xbsIndex_r4/Inverter  en=off  latency=0  dbl_ovrd=off  block_type=inv
  Inverter1 : xbsIndex_r4/Inverter  en=off  latency=0  dbl_ovrd=off  block_type=inv
  Logical : xbsIndex_r4/Logical  logical_function=AND  inputs=6  en=off  latency=0  precision=Full  arith_type=Unsigned  n_bits=16  bin_pt=0  align_bp=on  dbl_ovrd=off  block_type=logical
  Sym : Outport
  wires:
  Logical/1 -> Sym/1
  Corr/1 -> Logical/1, Delay2/1
  Delay2/1 -> Inverter/1, Delay3/1
  Inverter/1 -> Logical/2
  Delay3/1 -> Logical/3, Delay4/1
  Inverter1/1 -> Logical/4
  Delay4/1 -> Inverter1/1, Delay5/1
  Delay5/1 -> Logical/5
  En/1 -> Logical/6

== system wlan_phy_rx_pmd/DSSS Rx/Sym Sync/Sym Sync Timeout ==
  Sym : Inport
  Det : Inport  Port=2
  Constant1 : xbsIndex_r4/Constant  const=20*6  gui_display_data_type=Fixed-point  arith_type=Unsigned  n_bits=8  bin_pt=0  preci_type=Single  exp_width=8  frac_width=24  explicit_period=off  period=1  equ=P=C  opselect=C  inp2=PCIN>>17  opr=+  inp1=P  carry=CIN  dbl_ovrd=off  block_type=constant
      settings: block_version=VER_STRING_GOES_HERE
  Counter1 : xbsIndex_r4/Counter  cnt_type=Free Running  cnt_to=Inf  operation=Up  start_count=0  cnt_by_val=1  arith_type=Unsigned  n_bits=8  bin_pt=0  load_pin=off  rst=on  en=on  explicit_period=off  period=1  dbl_ovrd=off  use_behavioral_HDL=off  implementation=Fabric  block_type=counter
  Down Sample1 : xbsIndex_r4/Down Sample  sample_ratio=8  sample_phase="Last Value of Frame  (most efficient)"  en=off  latency=1  dbl_ovrd=off  block_type=dsamp
  Inverter4 : xbsIndex_r4/Inverter  en=off  latency=0  dbl_ovrd=off  block_type=inv
      settings: xl_area=[0 0 0 0 0 0 0]  block_version=VER_STRING_GOES_HERE
  Logical5 : xbsIndex_r4/Logical  logical_function=AND  inputs=2  en=off  latency=0  precision=Full  arith_type=Unsigned  n_bits=16  bin_pt=0  align_bp=on  dbl_ovrd=off  block_type=logical
  Posedge : Subsystem
  Relational1 : xbsIndex_r4/Relational  mode=a>b  en=off  latency=0  dbl_ovrd=off  block_type=relational
  S-R Latch2 : Subsystem
  Timeout : Outport
  wires:
  Posedge/1(Q) -> S-R Latch2/1(S)
  S-R Latch2/1(Q) -> Down Sample1/1
  Down Sample1/1 -> Counter1/2
  Inverter4/1 -> Logical5/1
  Counter1/1 -> Relational1/1
  Constant1/1 -> Relational1/2
  Relational1/1 -> Logical5/2
  [DSSS_RX_RESET] -> Counter1/1, S-R Latch2/2(R)
  Sym/1 -> Inverter4/1
  Det/1 -> Posedge/1(D)
  Logical5/1 -> Timeout/1

== system wlan_phy_rx_pmd/Detect & Decode/De-Interleave & De-Puncture/Read Addr Gen ==
  note: This is a brute force de-interleaver- the ROM stores the
      | de-interleaving pattern for each mod scheme separately.
      | The 802.11 standard interleavers have enough structure that
      | a non-ROM implementation is possible. But this design will
      | enable other interleaving patterns without changes to logic.
  Block Wr Done : Inport
  mod_sel : Inport  Port=2
  Reset : Inport  Port=3
  SIGNAL sym : Inport  Port=4
  rate_sel : Inport  Port=5
  Bus Creator : BusCreator  Inputs=2
  Bus Creator1 : BusCreator  Inputs=2
  Bus Creator2 : BusCreator  Inputs=2
  Ctrl : Subsystem
  De-Interleave DP-ROM : Subsystem
  Delay{1..4} (4 alike) : xbsIndex_r4/Delay  rst=off  infoeditControl=Selection of Reset will increase slice count due to use of real FFs and instead of SRLs  en=off  latency=1  dbl_ovrd=off  reg_retiming=off  block_type=delay
  Gateway Out{1..6,8..11} (10 alike) : xbsIndex_r4/Gateway Out  inherit_from_input=off  hdl_port=off  timing_constraint=None  locs_specified=off  LOCs={}  UseAsDAC=off  DACChannel='1'  block_type=gatewayout
  Int Rd Addr Gen : Scope  NumInputPorts=8
      settings: TimeRange=9000  YMin=0~0~0~0~-1~0~41.76470588235293~-0.1  YMax=2~1~1~1000~1~1~60.58823529411768~1.1  SaveName=ScopeData12
  Logical5 : xbsIndex_r4/Logical  logical_function=OR  inputs=2  en=off  latency=0  precision=Full  arith_type=Unsigned  n_bits=16  bin_pt=0  align_bp=on  dbl_ovrd=off  block_type=logical
  S-R Latch1 : Subsystem
  Rd Addr A : Outport
  Rd Addr B : Outport  Port=2
  Skip A : Outport  Port=3
  Skip B : Outport  Port=4
  Vout : Outport  Port=5
  wires:
  Ctrl/5(Vout) -> Delay2/1
  Gateway Out4/1 -> Int Rd Addr Gen/3  "Count En"
  Gateway Out3/1 -> Int Rd Addr Gen/2  "Wr done"
  Gateway Out2/1 -> Int Rd Addr Gen/1  "Rate Sel"
  Gateway Out8/1 -> Int Rd Addr Gen/6  "Vout"
  Logical5/1 -> S-R Latch1/2(R), Ctrl/3(Reset)
  Block Wr Done/1 -> Delay3/1
  S-R Latch1/1(Q) -> Ctrl/4(En), Gateway Out4/1
  Reset/1 -> Logical5/2
  Delay4/1 -> Gateway Out9/1, Skip A/1
  Delay3/1 -> S-R Latch1/1(S)
  Ctrl/6(Done) -> Logical5/1, Gateway Out3/1
  Delay1/1 -> Gateway Out10/1, Skip B/1
  De-Interleave DP-ROM/1(Addr A) -> Rd Addr A/1, Gateway Out6/1
  De-Interleave DP-ROM/2(Addr B) -> Rd Addr B/1, Gateway Out11/1
  Bus Creator1/1 -> Int Rd Addr Gen/7  "Rd Addr AB"
  Gateway Out6/1 -> Bus Creator1/1
  Gateway Out11/1 -> Bus Creator1/2
  mod_sel/1 -> Ctrl/1(mod_sel)
  rate_sel/1 -> Ctrl/2(rate_sel), Gateway Out2/1
  Ctrl/1(Ind A) -> De-Interleave DP-ROM/1(Ind A), Gateway Out1/1
  Ctrl/2(Ind B) -> De-Interleave DP-ROM/2(Ind B), Gateway Out5/1
  Ctrl/3(Skip A) -> Delay4/1
  Ctrl/4(Skip B) -> Delay1/1
  Delay2/1 -> Vout/1, Gateway Out8/1
  Gateway Out9/1 -> Bus Creator/1
  Gateway Out10/1 -> Bus Creator/2
  Bus Creator/1 -> Int Rd Addr Gen/8  "Skip AB"
  Bus Creator2/1 -> Int Rd Addr Gen/4  "Rd Ind AB"
  Gateway Out1/1 -> Bus Creator2/1
  Gateway Out5/1 -> Bus Creator2/2
  SIGNAL sym/1 -> Ctrl/5(SIGNAL sym)

== system wlan_phy_rx_pmd/Detect & Decode/De-Interleave & De-Puncture/Write Addr Gen ==
  note: SIGNAL symbols are always 48-subcarrier BPSK 1/2. N_CBPS
      | for post-SIGNAL symbols depends on decoded RATE/MCS values.
      | "From" tag here comes from SIGNAL Decode (11a) or
      | HT-SIG Decode (11n) blocks post-decoder.
  tvalid : Inport
  Reset : Inport  Port=2
  SIGNAL Sym : Inport  Port=3
  AddSub : xbsIndex_r4/AddSub  mode=Addition  use_carryin=off  use_carryout=off  en=off  latency=0  precision=User Defined  arith_type=Unsigned  n_bits=ceil(log2(MAX_NCBPS))  bin_pt=0  quantization=Truncate  overflow=Wrap  dbl_ovrd=off  use_behavioral_HDL=off  hw_selection=Fabric  pipelined=off  block_type=addsub
  AddSub1 : xbsIndex_r4/AddSub  mode=Subtraction  use_carryin=off  use_carryout=off  en=off  latency=0  precision=Full  arith_type=Unsigned  n_bits=ceil(log2(MAX_NCBPS))  bin_pt=0  quantization=Truncate  overflow=Wrap  dbl_ovrd=off  use_behavioral_HDL=off  hw_selection=Fabric  pipelined=off  block_type=addsub
  Constant10 : xbsIndex_r4/Constant  const=NCBPS_11a_BPSK-1  gui_display_data_type=Fixed-point  arith_type=Unsigned  n_bits=ceil(log2(MAX_NCBPS))  bin_pt=0  preci_type=Single  exp_width=8  frac_width=24  explicit_period=off  period=1  equ=P=C  opselect=C  inp2=PCIN>>17  opr=+  inp1=P  carry=CIN  dbl_ovrd=off  block_type=constant
  Constant11 : xbsIndex_r4/Constant  const=1  gui_display_data_type=Fixed-point  arith_type=Unsigned  n_bits=1  bin_pt=0  preci_type=Single  exp_width=8  frac_width=24  explicit_period=off  period=1  equ=P=C  opselect=C  inp2=PCIN>>17  opr=+  inp1=P  carry=CIN  dbl_ovrd=off  block_type=constant
  Constant6 : xbsIndex_r4/Constant  const=1  gui_display_data_type=Fixed-point  arith_type=Unsigned  n_bits=1  bin_pt=0  preci_type=Single  exp_width=8  frac_width=24  explicit_period=off  period=1  equ=P=C  opselect=C  inp2=PCIN>>17  opr=+  inp1=P  carry=CIN  dbl_ovrd=off  block_type=constant
  Counter : xbsIndex_r4/Counter  cnt_type=Free Running  cnt_to=1  operation=Up  start_count=0  cnt_by_val=2  arith_type=Unsigned  n_bits=ceil(log2(MAX_NCBPS))  bin_pt=0  load_pin=off  rst=on  en=on  explicit_period=off  period=1  dbl_ovrd=off  use_behavioral_HDL=off  implementation=Fabric  block_type=counter
  DeInt Wr Addr : Scope  NumInputPorts=8
      settings: TimeRange=20000  YMin=0~-0.1~0~0~0~-1~-1~-1  YMax=1~1.1~100~1~1~1~1~1  SaveName=ScopeData6
  Delay : xbsIndex_r4/Delay  rst=off  infoeditControl=Selection of Reset will increase slice count due to use of real FFs and instead of SRLs  en=off  latency=1  dbl_ovrd=off  reg_retiming=off  block_type=delay
  Gateway Out{1..6} (6 alike) : xbsIndex_r4/Gateway Out  inherit_from_input=off  hdl_port=off  timing_constraint=None  locs_specified=off  LOCs={}  UseAsDAC=off  DACChannel='1'  block_type=gatewayout
  Logical1 : xbsIndex_r4/Logical  logical_function=AND  inputs=2  en=off  latency=0  precision=Full  arith_type=Unsigned  n_bits=16  bin_pt=0  align_bp=on  dbl_ovrd=off  block_type=logical
  Logical5 : xbsIndex_r4/Logical  logical_function=OR  inputs=2  en=off  latency=0  precision=Full  arith_type=Unsigned  n_bits=16  bin_pt=0  align_bp=on  dbl_ovrd=off  block_type=logical
  Mux4 : xbsIndex_r4/Mux  inputs=2  en=off  latency=0  precision=Full  arith_type=Unsigned  n_bits=16  bin_pt=14  quantization=Truncate  overflow=Wrap  dbl_ovrd=off  block_type=mux
  Relational1 : xbsIndex_r4/Relational  mode=a>=b  en=off  latency=0  dbl_ovrd=off  block_type=relational
  Wr Addr A : Outport
  Wr Addr B : Outport  Port=2
  Wr En : Outport  Port=3
  Block Wr Done : Outport  Port=4
  wires:
  Logical5/1 -> Counter/1, Gateway Out2/1
  Counter/1 -> Relational1/1, AddSub/2, Wr Addr A/1, Gateway Out4/1
  tvalid/1 -> Counter/2, Gateway Out3/1, Wr En/1, Gateway Out1/1, Delay/1
  Relational1/1 -> Logical5/2, Logical1/1
  Reset/1 -> Logical5/1
  Gateway Out2/1 -> DeInt Wr Addr/1  "Reset"
  Gateway Out3/1 -> DeInt Wr Addr/2  "Valid In"
  Gateway Out4/1 -> DeInt Wr Addr/3  "Wr Addr A"
  Gateway Out1/1 -> DeInt Wr Addr/5  "Wr En"
  Gateway Out5/1 -> DeInt Wr Addr/6  "Block Done"
  Constant6/1 -> AddSub/1
  AddSub/1 -> Wr Addr B/1, Gateway Out6/1
  Gateway Out6/1 -> DeInt Wr Addr/4  "Wr Addr B"
  Mux4/1 -> Relational1/2
  SIGNAL Sym/1 -> Mux4/1
  Constant10/1 -> Mux4/3
  Constant11/1 -> AddSub1/2
  [OFDM_RX_DATA_N_CBPS] -> AddSub1/1
  AddSub1/1 -> Mux4/2
  Logical1/1 -> Block Wr Done/1, Gateway Out5/1
  Delay/1 -> Logical1/2

== system wlan_phy_rx_pmd/Detect & Decode/Decode/Byte Count ==
  Byte Valid : Inport
  Decode Start : Inport  Port=2
  Last Byte Ind : Inport  Port=3
  Byte Counter : xbsIndex_r4/Counter  cnt_type=Free Running  cnt_to=1  operation=Up  start_count=0  cnt_by_val=1  arith_type=Unsigned  n_bits=ceil(log2(MAX_NUM_BYTES))  bin_pt=0  load_pin=off  rst=on  en=on  explicit_period=off  period=1  dbl_ovrd=off  use_behavioral_HDL=off  implementation=Fabric  block_type=counter
  Inverter1 : xbsIndex_r4/Inverter  en=off  latency=0  dbl_ovrd=off  block_type=inv
  Logical : xbsIndex_r4/Logical  logical_function=AND  inputs=2  en=off  latency=0  precision=Full  arith_type=Unsigned  n_bits=16  bin_pt=0  align_bp=on  dbl_ovrd=off  block_type=logical
  Logical1 : xbsIndex_r4/Logical  logical_function=OR  inputs=2  en=off  latency=0  precision=Full  arith_type=Unsigned  n_bits=16  bin_pt=0  align_bp=on  dbl_ovrd=off  block_type=logical
  Logical3 : xbsIndex_r4/Logical  logical_function=AND  inputs=2  en=off  latency=0  precision=Full  arith_type=Unsigned  n_bits=16  bin_pt=0  align_bp=on  dbl_ovrd=off  block_type=logical
  Relational2 : xbsIndex_r4/Relational  mode=a>=b  en=off  latency=0  dbl_ovrd=off  block_type=relational
  S-R Latch1 : Subsystem
  " Byte Valid" : Outport
  Byte Index : Outport  Port=2
  Last Byte : Outport  Port=3
  wires:
  Logical/1 -> Byte Counter/2, " Byte Valid"/1
  Byte Counter/1 -> Byte Index/1, Relational2/1
  Logical3/1 -> S-R Latch1/2(R), Last Byte/1
  Decode Start/1 -> S-R Latch1/1(S), Logical1/1
  S-R Latch1/1(Q) -> Logical/1, Inverter1/1
  Byte Valid/1 -> Logical/2, Logical3/1
  Relational2/1 -> Logical3/2
  Last Byte Ind/1 -> Relational2/2
  Inverter1/1 -> Logical1/2
  Logical1/1 -> Byte Counter/1

== system wlan_phy_rx_pmd/Detect & Decode/Decode/Control ==
  dec_vin : Inport
  Reset : Inport  Port=2
  Start : Inport  Port=3
  Num Bytes : Inport  Port=4
  dec_vout : Inport  Port=5
  AddSub : xbsIndex_r4/AddSub  mode=Addition  use_carryin=off  use_carryout=off  en=off  latency=0  precision=Full  arith_type=Unsigned  n_bits=16  bin_pt=14  quantization=Truncate  overflow=Wrap  dbl_ovrd=off  use_behavioral_HDL=off  hw_selection=Fabric  pipelined=off  block_type=addsub
  Coded Bit Counter : xbsIndex_r4/Counter  cnt_type=Free Running  cnt_to=1  operation=Up  start_count=0  cnt_by_val=1  arith_type=Unsigned  n_bits=3+ceil(log2(MAX_NUM_BYTES))  bin_pt=0  load_pin=off  rst=on  en=on  explicit_period=off  period=1  dbl_ovrd=off  use_behavioral_HDL=off  implementation=Fabric  block_type=counter
  Concat : xbsIndex_r4/Concat  num_inputs=2  dbl_ovrd=off  block_type=concat
  Constant1 : xbsIndex_r4/Constant  const=14  gui_display_data_type=Fixed-point  arith_type=Unsigned  n_bits=5  bin_pt=0  preci_type=Single  exp_width=8  frac_width=24  explicit_period=off  period=1  equ=P=C  opselect=C  inp2=PCIN>>17  opr=+  inp1=P  carry=CIN  dbl_ovrd=off  block_type=constant
  Constant2 : xbsIndex_r4/Constant  const=0  gui_display_data_type=Fixed-point  arith_type=Unsigned  n_bits=3  bin_pt=0  preci_type=Single  exp_width=8  frac_width=24  explicit_period=off  period=1  equ=P=C  opselect=C  inp2=PCIN>>17  opr=+  inp1=P  carry=CIN  dbl_ovrd=off  block_type=constant
  Convert : xbsIndex_r4/Convert  gui_display_data_type=Fixed-point  arith_type=Unsigned  n_bits=1  bin_pt=0  float_type=Single  exp_bits=8  fraction_bits=24  quantization=Truncate  overflow=Wrap  en=off  latency=0  dbl_ovrd=off  pipeline=off  block_type=convert
  Convert2 : xbsIndex_r4/Convert  gui_display_data_type=Fixed-point  arith_type=Unsigned  n_bits=1  bin_pt=0  float_type=Single  exp_bits=8  fraction_bits=24  quantization=Truncate  overflow=Wrap  en=off  latency=0  dbl_ovrd=off  pipeline=off  block_type=convert
  Convert7 : xbsIndex_r4/Convert  gui_display_data_type=Fixed-point  arith_type=Unsigned  n_bits=1  bin_pt=0  float_type=Single  exp_bits=8  fraction_bits=24  quantization=Truncate  overflow=Wrap  en=off  latency=0  dbl_ovrd=off  pipeline=off  block_type=convert
  Decoder Ctrl : Scope  NumInputPorts=8
      settings: TimeRange=10000  YMin=-1~-1~1998.8~0~11100~-1~-1~0  YMax=1~1~2209.2~1~11275~1~1~1  SaveName=ScopeData40
  Delay1 : xbsIndex_r4/Delay  rst=off  infoeditControl=Selection of Reset will increase slice count due to use of real FFs and instead of SRLs  en=off  latency=2  dbl_ovrd=off  reg_retiming=off  block_type=delay
  Delay2 : xbsIndex_r4/Delay  rst=off  infoeditControl=Selection of Reset will increase slice count due to use of real FFs and instead of SRLs  en=off  latency=2  dbl_ovrd=off  reg_retiming=off  block_type=delay
  Early Trace Ctrl : Subsystem
  Gateway Out{1..5,7..8,10} (8 alike) : xbsIndex_r4/Gateway Out  inherit_from_input=off  hdl_port=off  timing_constraint=None  locs_specified=off  LOCs={}  UseAsDAC=off  DACChannel='1'  block_type=gatewayout
  Inverter : xbsIndex_r4/Inverter  en=off  latency=0  dbl_ovrd=off  block_type=inv
  Logical1 : xbsIndex_r4/Logical  logical_function=AND  inputs=2  en=off  latency=0  precision=Full  arith_type=Unsigned  n_bits=16  bin_pt=0  align_bp=on  dbl_ovrd=off  block_type=logical
  Logical3 : xbsIndex_r4/Logical  logical_function=OR  inputs=2  en=off  latency=0  precision=Full  arith_type=Unsigned  n_bits=16  bin_pt=0  align_bp=on  dbl_ovrd=off  block_type=logical
  Relational2 : xbsIndex_r4/Relational  mode=a<b  en=off  latency=0  dbl_ovrd=off  block_type=relational
  negedge : Subsystem
  vin : Outport
  rst# : Outport  Port=2
  start : Outport  Port=3
  pkt_end : Outport  Port=4
  early_trace_1 : Outport  Port=5
  early_trace_2 : Outport  Port=6
  wires:
  dec_vin/1 -> Coded Bit Counter/2, Gateway Out3/1, Logical1/2
  Early Trace Ctrl/1(Early Trace 1) -> early_trace_1/1, Gateway Out5/1
  Start/1 -> Gateway Out1/1, Logical3/2, Delay1/1, Coded Bit Counter/1
  Coded Bit Counter/1 -> Gateway Out4/1, Relational2/1, Early Trace Ctrl/1(Byte Ind)
  Num Bytes/1 -> Concat/1, Gateway Out2/1
  Constant2/1 -> Concat/2
  Concat/1 -> AddSub/1
  Inverter/1 -> Convert/1
  Logical3/1 -> Inverter/1, Early Trace Ctrl/3(reset)
  Reset/1 -> Logical3/1, Gateway Out10/1
  Delay1/1 -> Convert7/1
  Convert/1 -> rst#/1
  Convert7/1 -> start/1
  Gateway Out10/1 -> Decoder Ctrl/1  "Reset"
  Gateway Out1/1 -> Decoder Ctrl/2  "Start"
  Gateway Out2/1 -> Decoder Ctrl/3  "Num Bytes"
  Gateway Out3/1 -> Decoder Ctrl/4  "Vin in"
  Gateway Out4/1 -> Decoder Ctrl/5  "Bit Counter"
  Gateway Out5/1 -> Decoder Ctrl/6  "Early Trace En"
  negedge/1(Q) -> Delay2/1
  Gateway Out7/1 -> Decoder Ctrl/7  "End"
  Logical1/1 -> Convert2/1
  Convert2/1 -> vin/1, Gateway Out8/1
  Gateway Out8/1 -> Decoder Ctrl/8  "Vin Out"
  Relational2/1 -> Logical1/1, negedge/1(D)
  Constant1/1 -> AddSub/2
  AddSub/1 -> Relational2/2
  Delay2/1 -> Gateway Out7/1, pkt_end/1
  Early Trace Ctrl/2(Early Trace 2) -> early_trace_2/1
  dec_vout/1 -> Early Trace Ctrl/2(dec_vout)
